simulink model "non_linear_MBS_PF_final"  (R2021b) (continued)
== system non_linear_MBS_PF_final/Comparison Exp1 == (continued)
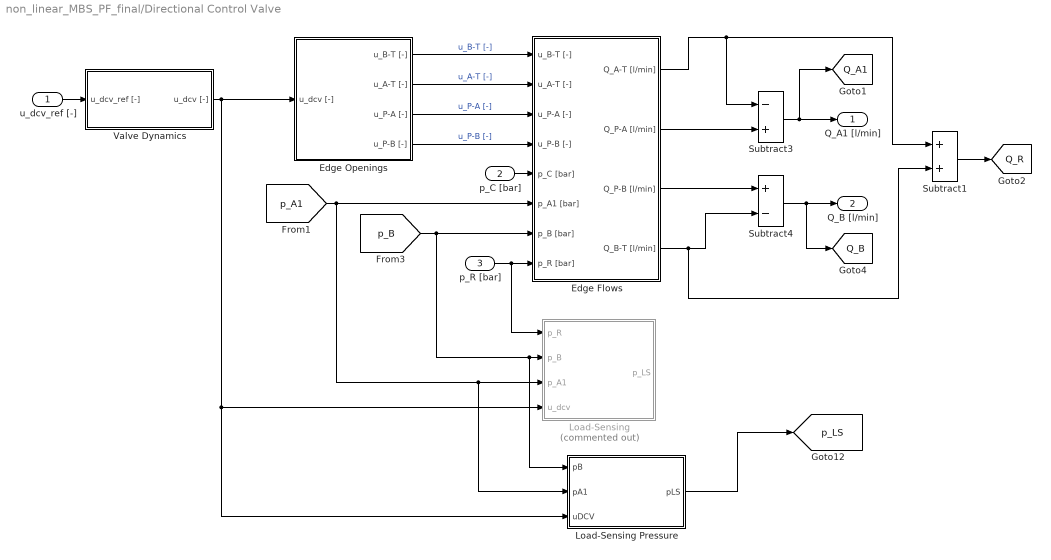
== system non_linear_MBS_PF_final/Directional Control Valve ==
  u_dcv_ref [-] : Inport
  p_C [bar] : Inport  Port=2
  p_R [bar] : Inport  Port=3
  Edge Flows : Subsystem
  Edge Openings : Subsystem
  Load-Sensing : MATLAB Function  Commented=on  TreatAsAtomicUnit=on
  Load-Sensing Pressure : Subsystem
  Subtract1 : Sum
  Subtract3 : Sum  Inputs=-+
  Subtract4 : Sum  Inputs=+-
  Valve Dynamics : Subsystem
  Q_A1 [l/min] : Outport  VectorParamsAs1DForOutWhenUnconnected=off
  Q_B [l/min] : Outport  Port=2  VectorParamsAs1DForOutWhenUnconnected=off
  tag scope: Q_A1=global, Q_B=global, Q_R=global, p_LS=global
  wires:
  Edge Flows/2(Q_P-A [l/min]) -> Subtract3/2
  u_dcv_ref [-]/1 -> Valve Dynamics/1(u_dcv_ref [-])
  p_R [bar]/1 -> Load-Sensing/1(p_R), Edge Flows/8(p_R [bar])
  [p_A1] -> Load-Sensing Pressure/2(pA1), Load-Sensing/3(p_A1), Edge Flows/6(p_A1 [bar])
  Subtract3/1 -> [Q_A1], Q_A1 [l/min]/1
  Subtract4/1 -> [Q_B], Q_B [l/min]/1
  [p_B] -> Load-Sensing Pressure/1(pB), Load-Sensing/2(p_B), Edge Flows/7(p_B [bar])
  p_C [bar]/1 -> Edge Flows/5(p_C [bar])
  Edge Flows/4(Q_B-T [l/min]) -> Subtract1/2, Subtract4/2
  Subtract1/1 -> [Q_R]
  Edge Openings/2(u_A-T [-]) -> Edge Flows/2(u_A-T [-])  "u_A-T [-]"
  Edge Openings/4(u_P-B [-]) -> Edge Flows/4(u_P-B [-])  "u_P-B [-]"
  Edge Openings/1(u_B-T [-]) -> Edge Flows/1(u_B-T [-])  "u_B-T [-]"
  Edge Openings/3(u_P-A [-]) -> Edge Flows/3(u_P-A [-])  "u_P-A [-]"
  Edge Flows/3(Q_P-B [l/min]) -> Subtract4/1
  Edge Flows/1(Q_A-T [l/min]) -> Subtract3/1, Subtract1/1
  Valve Dynamics/1(u_dcv [-]) -> Load-Sensing Pressure/3(uDCV), Load-Sensing/4(u_dcv), Edge Openings/1(u_dcv [-])
  Load-Sensing Pressure/1(pLS) -> [p_LS]

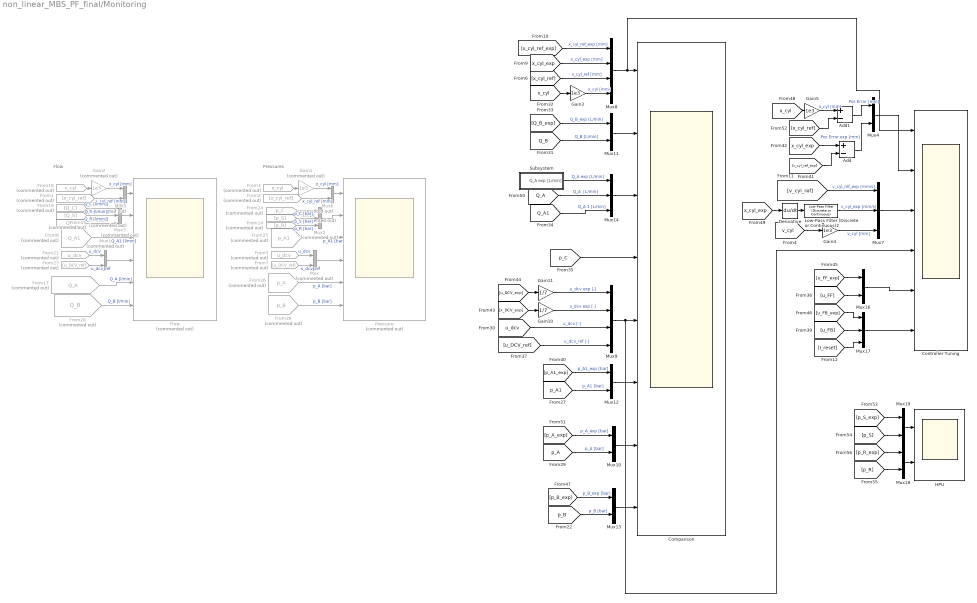
== system non_linear_MBS_PF_final/Monitoring ==
  note: Pressures
  note: Flow
  Add : Sum  Inputs=+-
  Add1 : Sum  Inputs=+-
  Comparison : Scope  NumInputPorts=8
  Controller Tuning : Scope  NumInputPorts=6
  Derivative : Derivative
  Flow : Scope  Commented=on  NumInputPorts=6
  From1 : From  Commented=on  GotoTag=x_cyl_ref  TagVisibility=global
  From13 : From  Commented=on  GotoTag=p_S  TagVisibility=global
  From14 : From  Commented=on  GotoTag=p_R  TagVisibility=global
  From15 : From  Commented=on  GotoTag=Q_S  TagVisibility=global
  From16 : From  Commented=on  GotoTag=Q_R  TagVisibility=global
  From17 : From  Commented=on  GotoTag=Q_A  TagVisibility=global
  From18 : From  Commented=on  GotoTag=x_cyl  TagVisibility=global
  From19 : From  Commented=on  GotoTag=Q_C  TagVisibility=global
  From2 : From  Commented=on  GotoTag=x_cyl_ref  TagVisibility=global
  From20 : From  Commented=on  GotoTag=Q_B  TagVisibility=global
  From21 : From  Commented=on  GotoTag=u_dcv  TagVisibility=global
  From23 : From  Commented=on  GotoTag=u_DCV_ref  TagVisibility=global
  From24 : From  Commented=on  GotoTag=p_C  TagVisibility=global
  From25 : From  Commented=on  GotoTag=p_A1  TagVisibility=global
  From26 : From  Commented=on  GotoTag=p_A  TagVisibility=global
  From28 : From  Commented=on  GotoTag=p_B  TagVisibility=global
  From3 : From  Commented=on  GotoTag=x_cyl  TagVisibility=global
  From5 : From  Commented=on  GotoTag=u_dcv  TagVisibility=global
  From7 : From  Commented=on  GotoTag=u_DCV_ref  TagVisibility=global
  From8 : From  Commented=on  GotoTag=Q_A1  TagVisibility=global
  Gain1 : Gain  Commented=on  Gain=1e3
  Gain10 : Gain  Gain=1/7
  Gain11 : Gain  Gain=1/7
  Gain2 : Gain  Commented=on  Gain=1e3
  Gain3 : Gain  Gain=1e3
  Gain4 : Gain  Gain=1e3
  Gain5 : Gain  Gain=1e3
  HPU : Scope  NumInputPorts=2
  Low-Pass Filter (Discrete or Continuous)2 : eeGeneralControl/Low-Pass Filter (Discrete or Continuous)  K=1  T=0.1  B=150  A=-150  init_option=Inherited from block input  x0=0  Ts=0
  Mux{,1,5..6} (4 alike) : Mux  Commented=on  Inputs=2
  Mux{4,10..13,16,18..19} (8 alike) : Mux  Inputs=2
  Mux14 : Mux  Inputs=3
  Mux17 : Mux  Inputs=3
  Mux2 : Mux  Commented=on  Inputs=3
  Mux3 : Mux  Commented=on  Inputs=3
  Mux7 : Mux  Inputs=3
  Mux8 : Mux
  Mux9 : Mux
  Pressure : Scope  Commented=on  NumInputPorts=6
  Subsystem : Subsystem
  wires:
  From28/1 -> Pressure/6  "p_B [bar]"
  From8/1 -> Flow/3  "Q_A1 [l/min]"
  From21/1 -> Mux1/1  "u_dcv"
  From17/1 -> Flow/5  "Q_A [l/min]"
  From26/1 -> Pressure/5  "p_A [bar]"
  From23/1 -> Mux1/2  "u_dcv_ref"
  From20/1 -> Flow/6  "Q_B [l/min]"
  Mux/1 -> Pressure/4
  From2/1 -> Mux6/2  "x_cyl_ref [mm]"
  From16/1 -> Mux3/3  "Q_R [l/min]]"
  From3/1 -> Gain1/1
  From24/1 -> Mux2/1  "p_C [bar]"
  From7/1 -> Mux/2  "u_dcv_ref"
  Mux1/1 -> Flow/4
  From25/1 -> Pressure/3  "p_A1 [bar]"
  Mux3/1 -> Flow/2
  Mux2/1 -> Pressure/2
  From5/1 -> Mux/1  "u_dcv"
  Mux5/1 -> Flow/1
  From14/1 -> Mux2/3  "p_R [bar]"
  From1/1 -> Mux5/2  "x_cyl_ref [mm]"
  From15/1 -> Mux3/2  "Q_S [l/min]]"
  From18/1 -> Gain2/1
  Gain2/1 -> Mux5/1  "x_cyl [mm]"
  From19/1 -> Mux3/1  "Q_C [l/min]]"
  From13/1 -> Mux2/2  "p_S [bar]"
  Gain1/1 -> Mux6/1  "x_cyl [mm]"
  Mux6/1 -> Pressure/1
  Mux8/1 -> Controller Tuning/1, Comparison/1
  [x_cyl_ref] -> Mux8/3  "x_cyl_ref [mm]"
  [x_cyl] -> Gain3/1
  Gain3/1 -> Mux8/4  "x_cyl [mm]"
  [x_cyl_ref_exp] -> Mux8/1  "x_cyl_ref_exp [mm]"
  [x_cyl_exp] -> Mux8/2  "x_cyl_exp [mm]"
  [Q_B] -> Mux11/2  "Q_B [L/min]"
  [Q_B_exp] -> Mux11/1  "Q_B_exp [L/min]"
  Mux11/1 -> Comparison/2
  [p_R] -> Mux18/2
  [p_R_exp] -> Mux18/1
  Mux18/1 -> HPU/2
  [p_S_exp] -> Mux19/1
  [p_S] -> Mux19/2
  Mux19/1 -> HPU/1
  [p_C] -> Comparison/4
  Mux17/1 -> Controller Tuning/6
  [u_FB] -> Mux17/2
  [u_FF] -> Mux16/2
  [u_FF_exp] -> Mux16/1
  [u_FB_exp] -> Mux17/1
  Mux16/1 -> Controller Tuning/5
  [x_cyl_exp] -> Add/1
  [x_cyl_ref] -> Add1/2
  [x_cyl_ref_exp] -> Add/2
  Gain5/1 -> Add1/1  "x_cyl [mm]"
  [x_cyl] -> Gain5/1
  Add/1 -> Mux4/2  "Pos Error exp [mm]"
  Add1/1 -> Mux4/1  "Pos Error [mm]"
  Mux4/1 -> Controller Tuning/2
  [I_reset] -> Mux17/3
  [x_DCV_exp] -> Gain10/1
  Gain10/1 -> Mux9/2  "x_dcv exp [-]"
  [u_DCV_ref] -> Mux9/4  "u_dcv_ref [-]"
  [u_dcv] -> Mux9/3  "u_dcv [-]"
  Gain11/1 -> Mux9/1  "u_dcv exp [-]"
  [p_A1_exp] -> Mux12/1  "p_A1_exp [bar]"
  [p_A1] -> Mux12/2  "p_A1 [bar]"
  Mux12/1 -> Comparison/6
  Mux9/1 -> Controller Tuning/4, Comparison/5
  Low-Pass Filter (Discrete or Continuous)2/1 -> Mux7/2  "v_cyl_exp [mm/s]"
  Mux7/1 -> Controller Tuning/3
  Derivative/1 -> Low-Pass Filter (Discrete or Continuous)2/1
  [v_cyl_ref] -> Mux7/1  "v_cyl_ref_exp [mm/s]"
  [v_cyl] -> Gain4/1
  [x_cyl_exp] -> Derivative/1
  Gain4/1 -> Mux7/3  "v_cyl [mm]"
  [Q_A1] -> Mux14/3  "Q_A 1 [L/min]"
  Subsystem/1(Q_A exp [L/min]) -> Mux14/1  "Q_A exp [L/min]"
  [Q_A] -> Mux14/2  "Q_A  [L/min]"
  Mux14/1 -> Comparison/3
  [u_DCV_exp] -> Gain11/1
  [p_B] -> Mux13/2  "p_B [bar]"
  [p_B_exp] -> Mux13/1  "p_B_exp [bar]"
  [p_A] -> Mux10/2  "p_A [bar]"
  [p_A_exp] -> Mux10/1  "p_A_exp [bar]"
  Mux13/1 -> Comparison/8
  Mux10/1 -> Comparison/7

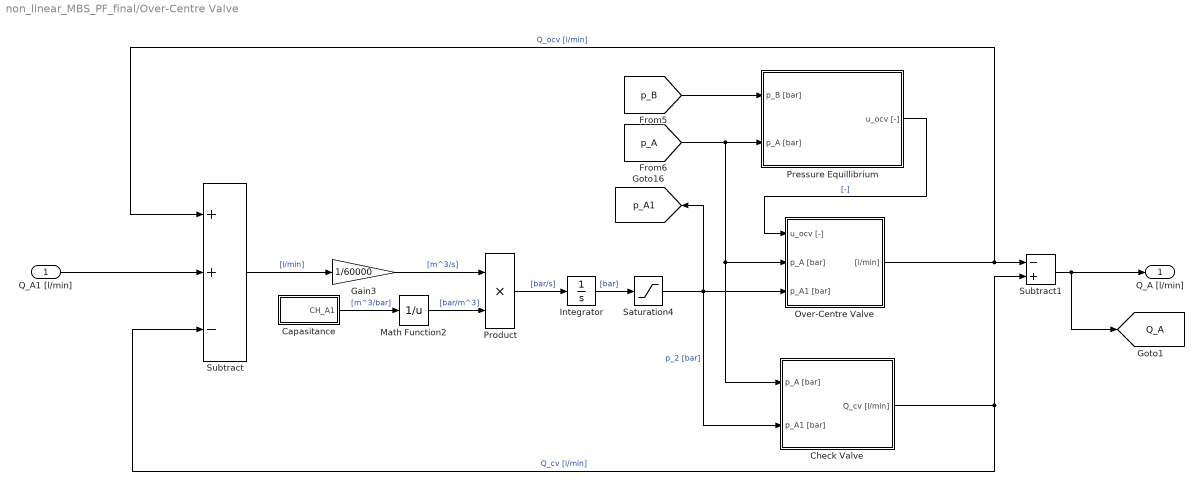
== system non_linear_MBS_PF_final/Over-Centre Valve ==
  Q_A1 [l/min] : Inport
  Capasitance : Subsystem
  Check Valve : Subsystem
  Gain3 : Gain  Gain=1/60000
  Integrator : Integrator  InitialCondition=p0_A1
  Math Function2 : Math  Operator=reciprocal  SignedPower=on  SaturateOnIntegerOverflow=on
  Over-Centre Valve : Subsystem
  Pressure Equillibrium : Subsystem
  Product : Product
  Saturation4 : Saturate  UpperLimit=200  LowerLimit=p_R  ZeroCross=off
  Subtract : Sum  Inputs=++-
  Subtract1 : Sum  Inputs=-+
  Q_A [l/min] : Outport  PortDimensions=1  VectorParamsAs1DForOutWhenUnconnected=off
  tag scope: Q_A=global, p_A1=global
  wires:
  [p_B] -> Pressure Equillibrium/1(p_B [bar])
  Pressure Equillibrium/1(u_ocv [-]) -> Over-Centre Valve/1(u_ocv [-])  "[-]"
  Check Valve/1(Q_cv [l/min]) -> Subtract/3, Subtract1/2  "Q_cv [l/min]"
  Over-Centre Valve/1("[l/min]") -> Subtract1/1, Subtract/1  "Q_ocv [l/min]"
  Saturation4/1 -> Check Valve/2(p_A1 [bar]), Over-Centre Valve/3(p_A1 [bar]), [p_A1]  "p_2 [bar]"
  Subtract/1 -> Gain3/1  "[l/min]"
  Q_A1 [l/min]/1 -> Subtract/2
  Product/1 -> Integrator/1  "[bar/s]"
  Integrator/1 -> Saturation4/1  "[bar]"
  Gain3/1 -> Product/1  "[m^3/s]"
  Subtract1/1 -> [Q_A], Q_A [l/min]/1
  [p_A] -> Pressure Equillibrium/2(p_A [bar]), Over-Centre Valve/2(p_A [bar]), Check Valve/1(p_A [bar])
  Capasitance/1("CH_A1 ") -> Math Function2/1  "[m^3/bar]"
  Math Function2/1 -> Product/2  "[bar/m^3]"

== system non_linear_MBS_PF_final/Pressure Compensator1 ==
  p_S [bar] : Inport
  Compensator From Morten Bak Paper : MATLAB Function  TreatAsAtomicUnit=on
  "Compensator setting [bar] " : Constant  Value=p_pc_set
  Transfer Fcn1 : TransferFcn  Denominator=[tau_pc 1]
  p_C [bar] : Outport  VectorParamsAs1DForOutWhenUnconnected=off
  tag scope: p_C=global
  wires:
  [p_LS] -> Compensator From Morten Bak Paper/3(p_LS)
  "Compensator setting [bar] "/1 -> Compensator From Morten Bak Paper/1(p0)
  p_S [bar]/1 -> Compensator From Morten Bak Paper/2(p_S)
  Compensator From Morten Bak Paper/1(p_C) -> Transfer Fcn1/1  "p_C [bar]"
  Transfer Fcn1/1 -> [p_C], p_C [bar]/1  "u_ocv_ref [-]"

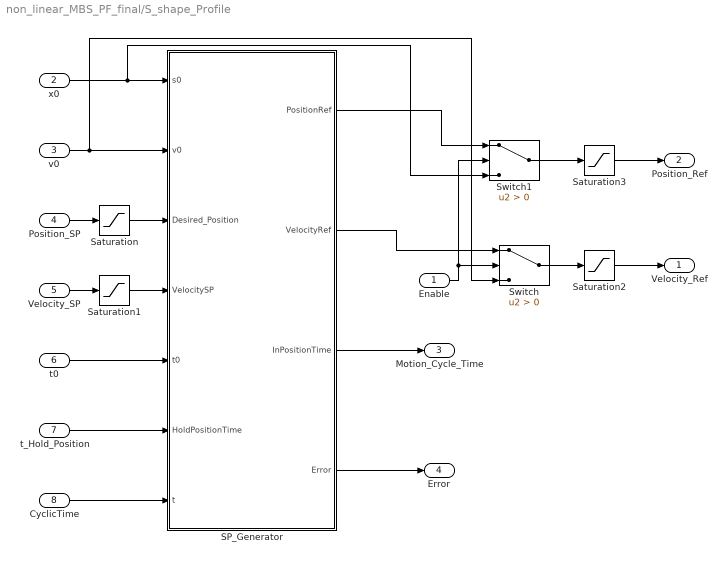
== system non_linear_MBS_PF_final/S_shape_Profile ==
  Enable : Inport  OutDataTypeStr=boolean
  x0 : Inport  Port=2
  v0 : Inport  Port=3
  Position_SP : Inport  Port=4
  Velocity_SP : Inport  Port=5
  t0 : Inport  Port=6
  t_Hold_Position : Inport  Port=7
  CyclicTime : Inport  Port=8
  SP_Generator : MATLAB Function  TreatAsAtomicUnit=on
  Saturation : Saturate  UpperLimit=500  LowerLimit=0
  Saturation1 : Saturate  UpperLimit=150  LowerLimit=-150
  Saturation2 : Saturate  UpperLimit=150  LowerLimit=-150
  Saturation3 : Saturate  UpperLimit=500  LowerLimit=0
  Switch : Switch  Criteria=u2 > Threshold
  Switch1 : Switch  Criteria=u2 > Threshold
  Velocity_Ref : Outport  VectorParamsAs1DForOutWhenUnconnected=off
  Position_Ref : Outport  Port=2  VectorParamsAs1DForOutWhenUnconnected=off
  Motion_Cycle_Time : Outport  Port=3  VectorParamsAs1DForOutWhenUnconnected=off
  Error : Outport  Port=4  VectorParamsAs1DForOutWhenUnconnected=off
  wires:
  Saturation/1 -> SP_Generator/3(Desired_Position)
  Saturation1/1 -> SP_Generator/4(VelocitySP)
  t0/1 -> SP_Generator/5(t0)
  t_Hold_Position/1 -> SP_Generator/6(HoldPositionTime)
  Velocity_SP/1 -> Saturation1/1
  SP_Generator/3(InPositionTime) -> Motion_Cycle_Time/1
  CyclicTime/1 -> SP_Generator/7(t)
  SP_Generator/4(Error) -> Error/1
  Position_SP/1 -> Saturation/1
  Saturation2/1 -> Velocity_Ref/1
  Switch/1 -> Saturation2/1
  x0/1 -> Switch1/3, SP_Generator/1(s0)
  SP_Generator/1(PositionRef) -> Switch1/1
  Saturation3/1 -> Position_Ref/1
  v0/1 -> Switch/3, SP_Generator/2(v0)
  Enable/1 -> Switch1/2, Switch/2
  SP_Generator/2(VelocityRef) -> Switch/1
  Switch1/1 -> Saturation3/1

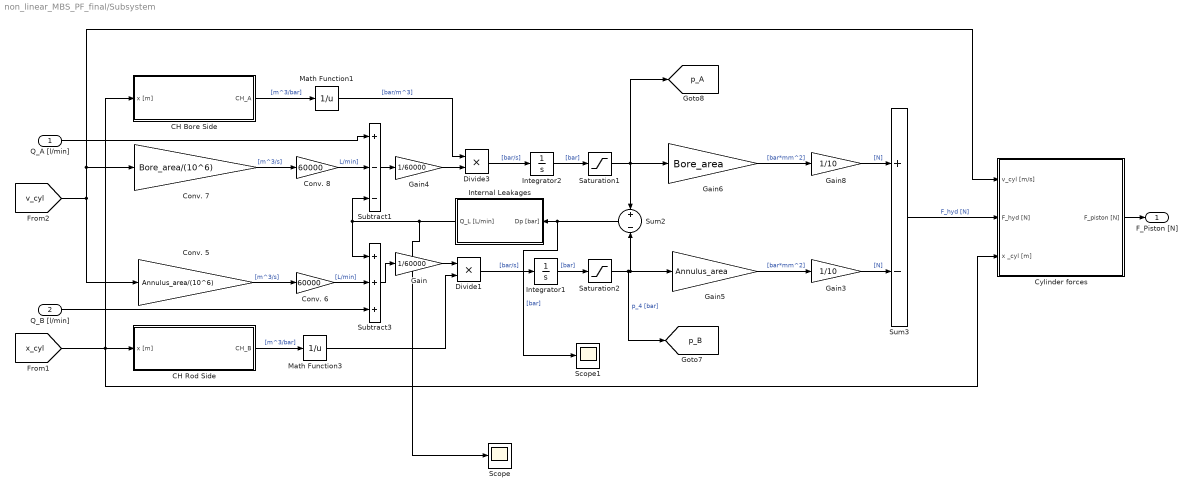
== system non_linear_MBS_PF_final/Subsystem ==
  Q_A [l/min] : Inport
  Q_B [l/min] : Inport  Port=2
  CH Bore Side : Subsystem
  CH Rod Side : Subsystem
  Conv. 5 : Gain  Gain=Annulus_area/(10^6)
  Conv. 6 : Gain  Gain=60000
  Conv. 7 : Gain  Gain=Bore_area/(10^6)
  Conv. 8 : Gain  Gain=60000
  Cylinder forces : Subsystem
  Divide1 : Product  Inputs=**
  Divide3 : Product  Inputs=**
  Gain : Gain  Gain=1/60000
  Gain3 : Gain  Gain=1/10
  Gain4 : Gain  Gain=1/60000
  Gain5 : Gain  Gain=Annulus_area
  Gain6 : Gain  Gain=Bore_area
  Gain8 : Gain  Gain=1/10
  Integrator1 : Integrator  InitialCondition=p0_B  LowerSaturationLimit=0
  Integrator2 : Integrator  InitialCondition=p0_A  LowerSaturationLimit=0
  Internal Leakages : Subsystem
  Math Function1 : Math  Operator=reciprocal  SignedPower=on  SaturateOnIntegerOverflow=on
  Math Function3 : Math  Operator=reciprocal  SignedPower=on  SaturateOnIntegerOverflow=on
  Saturation1 : Saturate  UpperLimit=200  LowerLimit=p_R  ZeroCross=off
  Saturation2 : Saturate  UpperLimit=200  LowerLimit=p_R  ZeroCross=off
  Scope : Scope
  Scope1 : Scope
  Subtract1 : Sum  Inputs=+--
  Subtract3 : Sum  Inputs=+++
  Sum2 : Sum  Inputs=+-
  Sum3 : Sum  Inputs=+-
  F_Piston [N] : Outport  VectorParamsAs1DForOutWhenUnconnected=off
  tag scope: p_A=global, p_B=global
  wires:
  Sum3/1 -> Cylinder forces/2(F_hyd [N])  "F_hyd [N]"
  Gain8/1 -> Sum3/1  "[N]"
  Gain3/1 -> Sum3/2  "[N]"
  Saturation1/1 -> Gain6/1, [p_A], Sum2/1
  Gain5/1 -> Gain3/1  "[bar*mm^2]"
  Gain6/1 -> Gain8/1  "[bar*mm^2]"
  Cylinder forces/1(F_piston [N]) -> F_Piston [N]/1
  [v_cyl] -> Cylinder forces/1(v_cyl [m/s]), Conv. 7/1, Conv. 5/1
  [x_cyl] -> CH Rod Side/1(x [m]), CH Bore Side/1(x [m]), Cylinder forces/3(x _cyl [m])
  Integrator1/1 -> Saturation2/1  "[bar]"
  Q_B [l/min]/1 -> Subtract3/3
  Divide1/1 -> Integrator1/1  "[bar/s]"
  Saturation2/1 -> Sum2/2, Gain5/1, [p_B]  "p_4 [bar]"
  Gain/1 -> Divide1/1
  Integrator2/1 -> Saturation1/1  "[bar]"
  Gain4/1 -> Divide3/2
  Divide3/1 -> Integrator2/1  "[bar/s]"
  Math Function1/1 -> Divide3/1  "[bar/m^3]"
  CH Bore Side/1(CH_A) -> Math Function1/1  "[m^3/bar]"
  Math Function3/1 -> Divide1/2
  CH Rod Side/1(CH_B) -> Math Function3/1  "[m^3/bar]"
  Subtract3/1 -> Gain/1
  Conv. 6/1 -> Subtract3/2  "[L/min]"
  Conv. 5/1 -> Conv. 6/1  "[m^3/s]"
  Subtract1/1 -> Gain4/1
  Q_A [l/min]/1 -> Subtract1/1
  Conv. 7/1 -> Conv. 8/1  "[m^3/s]"
  Conv. 8/1 -> Subtract1/2  "L/min]"
  Sum2/1 -> Scope1/1, Internal Leakages/1(Dp [bar])  "[bar]"
  Internal Leakages/1(Q_L [L/min]) -> Scope/1, Subtract3/1, Subtract1/3

== system non_linear_MBS_PF_final/Subsystem1 ==
  Clock : Clock
  Constant1 : Constant  Value=tWait
  Constant15 : Constant  Value=uS_sp
  Constant2 : Constant  Value=t0
  Constant3 : Constant  Value=uS0
  Constant4 : Constant  Value=tStepUp
  Constant5 : Constant  OutDataTypeStr=boolean
  Constant6 : Constant  Value=tStepDown
  Constant7 : Constant  Value=DB
  Display : Display
  Gain20 : Gain  Gain=-1
  VCC_Step_Function_Validation : Subsystem  TreatAsAtomicUnit=on
  u_Ref [-] : Outport  VectorParamsAs1DForOutWhenUnconnected=off
  tag scope: uV_sp=global
  wires:
  VCC_Step_Function_Validation/2(EndTime) -> Display/1
  Constant4/1 -> VCC_Step_Function_Validation/7(tHold1)
  Constant1/1 -> VCC_Step_Function_Validation/8(tStep)
  Clock/1 -> VCC_Step_Function_Validation/5(CyclicTime)
  Constant2/1 -> VCC_Step_Function_Validation/6(t0)
  Constant15/1 -> VCC_Step_Function_Validation/2(u_SP)
  VCC_Step_Function_Validation/1(u_ref) -> Gain20/1  "pB_tfs [Pa]"
  Constant3/1 -> VCC_Step_Function_Validation/3(uS0)
  Constant6/1 -> VCC_Step_Function_Validation/9(tHold2)
  Constant5/1 -> VCC_Step_Function_Validation/1(Enable)
  Constant7/1 -> VCC_Step_Function_Validation/4(DB)
  Gain20/1 -> [uV_sp], u_Ref [-]/1  "xS"

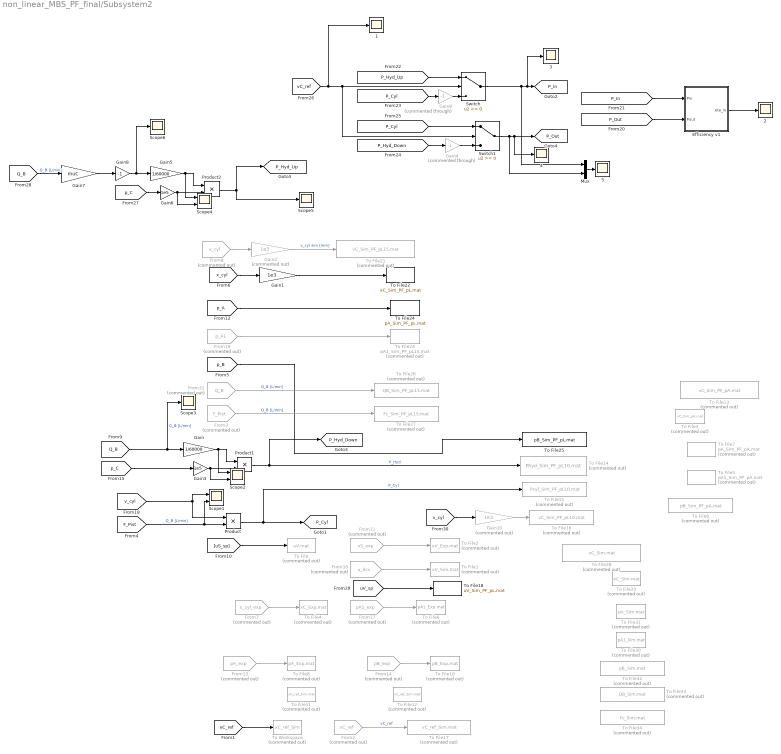
== system non_linear_MBS_PF_final/Subsystem2 ==
  {1..5} (5 alike) : Scope
  Efficiency v1 : Subsystem
  From11 : From  Commented=on  GotoTag=xS_exp  TagVisibility=global
  From13 : From  Commented=on  GotoTag=pA_exp  TagVisibility=global
  From14 : From  Commented=on  GotoTag=pB_exp  TagVisibility=global
  From16 : From  Commented=on  GotoTag=u_dcv  TagVisibility=global
  From17 : From  Commented=on  GotoTag=pA1_exp  TagVisibility=global
  From19 : From  Commented=on  GotoTag=p_A1  TagVisibility=global
  From2 : From  Commented=on  GotoTag=vC_ref  TagVisibility=global
  From3 : From  Commented=on  GotoTag=F_Pist  TagVisibility=global
  From31 : From  Commented=on  GotoTag=Q_B  TagVisibility=global
  From7 : From  Commented=on  GotoTag=x_cyl_exp  TagVisibility=global
  From8 : From  Commented=on  GotoTag=v_cyl  TagVisibility=global
  Gain : Gain  Gain=1/60000
  Gain1 : Gain  Gain=1e3
  Gain10 : Gain  Commented=on  Gain=1e3
  Gain2 : Gain  Commented=on  Gain=1e3
  Gain3 : Gain  Gain=1e5
  Gain4 : Gain  Commented=through  Gain=-1
  Gain5 : Gain  Gain=1/60000
  Gain6 : Gain  Gain=1e5
  Gain7 : Gain  Gain=muC
  Gain8 : Gain  Gain=-1
  Gain9 : Gain  Commented=through  Gain=-1
  Mux : Mux  Inputs=2
  Product : Product
  Product1 : Product
  Product2 : Product
  Scope1 : Scope  NumInputPorts=2
  Scope2 : Scope  NumInputPorts=2
  Scope3 : Scope
  Scope4 : Scope  NumInputPorts=2
  Scope5 : Scope
  Scope6 : Scope
  Switch : Switch
  Switch1 : Switch
  To File : ToFile  Commented=on  Filename=uV.mat  MatrixName=uV  SaveFormat=Timeseries
  To File1 : ToFile  Commented=on  Filename=xV_Sim.mat  MatrixName=xV_Sim  SaveFormat=Timeseries
  To File10 : ToFile  Commented=on  Filename=pB_Exp.mat  MatrixName=pB_Exp  SaveFormat=Timeseries
  To File11 : ToFile  Commented=on  Filename=xC_ref_Sim.mat  MatrixName=xC_ref_Sim  SaveFormat=Timeseries
  To File12 : ToFile  Commented=on  Filename=vC_ref_Sim.mat  MatrixName=vC_Sim  SaveFormat=Timeseries
  To File13 : ToFile  Commented=on  Filename=vC_Sim_PF_pA.mat  MatrixName=vC_Sim_PF_pA  SaveFormat=Timeseries
  To File14 : ToFile  Commented=on  Filename=Phyd_Sim_PF_pL10.mat  MatrixName=pB_Sim_PF_pL10  SaveFormat=Timeseries
  To File15 : ToFile  Commented=on  Filename=Pcyl_Sim_PF_pL10.mat  MatrixName=Pcyl_Sim_PF_pL10  SaveFormat=Timeseries
  To File16 : ToFile  Commented=on  Filename=vC_Sim_PF_pL10.mat  MatrixName=vC_Sim_PF_pL10  SaveFormat=Timeseries
  To File17 : ToFile  Commented=on  Filename=vC_ref_Sim.mat  MatrixName=vC_ref_Sim  SaveFormat=Timeseries
  To File18 : ToFile  Filename=uV_Sim_PF_pL.mat  MatrixName=uV_Sim_PF_pL  SaveFormat=Timeseries
  To File2 : ToFile  Commented=on  Filename=xV_Exp.mat  MatrixName=xV_Exp  SaveFormat=Timeseries
  To File21 : ToFile  Commented=on  Filename=vC_Sim_PF_pL15.mat  MatrixName=vC_Sim_PF_pL15  SaveFormat=Timeseries
  To File22 : ToFile  Filename=xC_Sim_PF_pL.mat  MatrixName=xC_Sim_PF_pL  SaveFormat=Timeseries
  To File23 : ToFile  Commented=on  Filename=pA1_Sim_PF_pL15.mat  MatrixName=pA1_Sim_PF_pL15  SaveFormat=Timeseries
  To File24 : ToFile  Filename=pA_Sim_PF_pL.mat  MatrixName=pA_Sim_PF_pL  SaveFormat=Timeseries
  To File25 : ToFile  Filename=pB_Sim_PF_pL.mat  MatrixName=pB_Sim_PF_pL  SaveFormat=Timeseries
  To File26 : ToFile  Commented=on  Filename=QB_Sim_PF_pL15.mat  MatrixName=QB_Sim_PF_pL15  SaveFormat=Timeseries
  To File27 : ToFile  Commented=on  Filename=Fc_Sim_PF_pL15.mat  MatrixName=Fc_Sim_PF_pL15  SaveFormat=Timeseries
  To File28 : ToFile  Commented=on  Filename=vC_Sim.mat  MatrixName=vC_Sim  SaveFormat=Timeseries
  To File29 : ToFile  Commented=on  Filename=xC_Sim.mat  MatrixName=xC_Sim  SaveFormat=Timeseries
  To File3 : ToFile  Commented=on  Filename=xC_Sim_pA.mat  MatrixName=xC_Sim_pA  SaveFormat=Timeseries
  To File30 : ToFile  Commented=on  Filename=pA1_Sim.mat  MatrixName=pA1_Sim  SaveFormat=Timeseries
  To File31 : ToFile  Commented=on  Filename=pA_Sim.mat  MatrixName=pA_Sim  SaveFormat=Timeseries
  To File32 : ToFile  Commented=on  Filename=pB_Sim.mat  MatrixName=pB_Sim  SaveFormat=Timeseries
  To File33 : ToFile  Commented=on  Filename=QB_Sim.mat  MatrixName=QB_Sim  SaveFormat=Timeseries
  To File34 : ToFile  Commented=on  Filename=Fc_Sim.mat  MatrixName=Fc_Sim  SaveFormat=Timeseries
  To File4 : ToFile  Commented=on  Filename=xC_Exp.mat  MatrixName=xC_Exp  SaveFormat=Timeseries
  To File5 : ToFile  Commented=on  Filename=pA1_Sim_PF_pA.mat  MatrixName=pA1_Sim_PF_pA  SaveFormat=Timeseries
  To File6 : ToFile  Commented=on  Filename=pA1_Exp.mat  MatrixName=pA1_Exp  SaveFormat=Timeseries
  To File7 : ToFile  Commented=on  Filename=pA_Sim_PF_pA.mat  MatrixName=pA_Sim_PF_pA  SaveFormat=Timeseries
  To File8 : ToFile  Commented=on  Filename=pA_Exp.mat  MatrixName=pA_Exp  SaveFormat=Timeseries
  To File9 : ToFile  Commented=on  Filename=pB_Sim_PF_pA.mat  MatrixName=pB_Sim_PF_pA  SaveFormat=Timeseries
  To Workspace : ToWorkspace  Commented=on  VariableName=xC_ref_Sim  SampleTime=0.001
      settings: Save2DSignal=2-D array (concatenate along first dimension)
  tag scope: P_Cyl=global, P_Hyd_Down=global, P_Hyd_Up=global, P_In=global, P_Out=global
  wires:
  [x_cyl] -> Gain1/1
  [uS_sp] -> To File/1
  From11/1 -> To File2/1
  From16/1 -> To File1/1
  From7/1 -> To File4/1
  From17/1 -> To File6/1
  From13/1 -> To File8/1
  From14/1 -> To File10/1
  From8/1 -> Gain2/1
  Gain2/1 -> To File21/1  "v_cyl sim [mm]"
  [p_B] -> To File25/1
  From19/1 -> To File23/1
  [p_A] -> To File24/1
  Gain1/1 -> To File22/1
  From31/1 -> To File26/1  "Q_B [L/min]"
  From3/1 -> To File27/1  "Q_B [L/min]"
  [F_Pist] -> Scope1/2, Product/2  "Q_B [L/min]"
  [Q_B] -> Scope3/1, Gain/1  "Q_B [L/min]"
  Gain/1 -> Scope2/1, Product1/1
  Gain3/1 -> Scope2/2, Product1/2
  [p_C] -> Gain3/1
  [v_cyl] -> Product/1, Scope1/1
  Product1/1 -> [P_Hyd_Down], To File14/1  "P_Hyd"
  [P_Hyd_Up] -> Switch/1
  [P_In] -> Efficiency v1/1(Pin)
  Switch/1 -> Mux/1, [P_In], 3/1
  [P_Out] -> Efficiency v1/2(Pout)
  [vC_ref] -> 1/1, Switch/2, Switch1/2
  Switch1/1 -> Mux/2, 4/1, [P_Out]
  [P_Hyd_Down] -> Gain4/1
  Efficiency v1/1(eta_io) -> 2/1
  Product/1 -> To File15/1, [P_Cyl]  "P_Cyl"
  [P_Cyl] -> Switch1/1
  [P_Cyl] -> Gain9/1
  [Q_B] -> Gain7/1  "Q_B [L/min]"
  Product2/1 -> [P_Hyd_Up], Scope5/1
  Gain5/1 -> Scope4/1, Product2/1
  Gain8/1 -> Scope6/1, Gain5/1
  Gain6/1 -> Scope4/2, Product2/2
  Gain7/1 -> Gain8/1
  [p_C] -> Gain6/1
  Gain4/1 -> Switch1/3
  Gain9/1 -> Switch/3
  Mux/1 -> 5/1
  [xC_ref] -> To Workspace/1
  From2/1 -> To File17/1  "vC_ref"
  Gain10/1 -> To File16/1
  [v_cyl] -> Gain10/1
  [uV_sp] -> To File18/1
